simulink model "ACC_scenario_003_cs"  (Simulink 7.6)
settings: Solver=ode5  SolverMode=SingleTasking  StartTime=0.0  StopTime=inf  FixedStep=1/100  RelTol=1e-3
model workspace (MAT data in the model file):
  mbox_table : struct (value not decoded)
  model_closed = 0
  sim_status = 0
PreLoadFcn:
  | check_NUTS_defined;
  | 
  | assignin('base',['model_closed_' bdroot],false);addpath('../includes_ACC');
InitFcn:
  | PreScanExperimentInitFcn(bdroot)
StopFcn:
  | PreScanExperimentStopFcn(bdroot)
undefined symbols (not defined in the file): DiscIntNeverNeededParam, x_ini_VRML, y_ini_VRML, z_ini_VRML
libraries: PreScanSimulinkLibrary x55, simulink x26, PreScanUsefulBlocks x17, simulink_extras x15, simulink_need_slupdate x1
(part 1 of 28)
CloseFcn:
  | evalin('base',['clear model_closed_' bdroot]);
settings Model: Creator=PRESCAN  LastModifiedBy=buildbot
settings OptimizationCC: InitFltsAndDblsToZero=on
settings DebuggingCC: SaveWithDisabledLinksMsg=none  SaveWithParameterizedLinksMsg=none  SignalResolutionControl=TryResolveAllWithWarning  SolverPrmCheckMsg=none  MultiTaskDSMMsg=warning  MultiTaskCondExecSysMsg=none
settings SFSimCC: SimParseCustomCode=off
settings RTWCC: IncludeERTFirstTime=on
settings GRTTargetCC: TargetFcnLib=ansi_tfl_tmw.mat

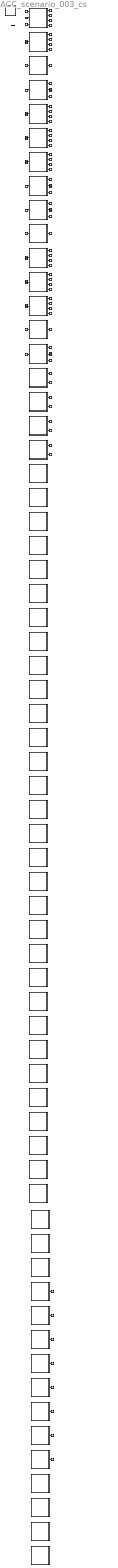
== system ACC_scenario_003_cs ==
  note: Demo Help
  note: Regenerate
  note: Record for Replay
  note: Open Useful Blocks
  note: PreScan Options
  note: Version:
  note: 7.4.0
  note: Author(s):
  note: TASS International
  note: Descriptive text:
  note: ACC test scenario
  note: ID
      |
      | 1
      | 3
      | 5
      | 383
      | 385
      | 386
      | 387
      | 388
      | 389
      | 390
      | 391
      | 392
      | 393
      | 724
      | 728
      | 380
      | 382
      | 381
      | 384
      | 403
      | 405
      | 407
      | 408
      | 409
      | 410
      | 411
      | 412
      | 413
      | 414
      | 415
      | 416
      | 417
      | 418
      | 419
      | 420
      | 421
      | 436
      | 437
      | 438
      | 439
      | 440
      | 441
      | 442
      | 443
      | 444
      | 445
      | 446
      | 447
      | 448
      | 449
      | 450
      | 451
      | 452
      | 628
      | 629
      | 612
      | 631
      | 630
      | 633
      | 632
      | 635
      | 634
      | 637
      | 636
      | 639
      | 640
      | 641
      | 642
      | 643
      | 644
      | 645
      | 716
      | 717
      | 718
      | 719
      | 592
      | 613
  note: OBU-Beacon ID
  note: TypeID
      |
      | 1
      | 1
      | 5
      | 2
      | 1
      | 1
      | 3
      | 3
      | 3
      | 7
      | 1
      | 1
      | 1
      | 3
      | 3
      | 15
      | 15
      | 15
      | 15
      | 15
      | 15
      | 15
      | 15
      | 15
      | 15
      | 15
      | 15
      | 15
      | 15
      | 15
      | 15
      | 15
      | 15
      | 15
      | 15
      | 15
      | 15
      | 15
      | 15
      | 15
      | 15
      | 15
      | 15
      | 15
      | 15
      | 15
      | 15
      | 15
      | 15
      | 15
      | 15
      | 15
      | 15
      | 15
      | 15
      | 15
      | 15
      | 15
      | 15
      | 15
      | 15
      | 15
      | 15
      | 15
      | 15
      | 15
      | 15
      | 15
      | 15
      | 15
      | 15
      | 15
      | 15
      | 15
      | 15
      | 15
      | 15
  note: TypeTag
      |
      | Car
      | Car
      | Cal element
      | Motor
      | Car
      | Car
      | Truck\Bus
      | Truck\Bus
      | Truck\Bus
      | Unknown
      | Car
      | Car
      | Car
      | Truck\Bus
      | Truck\Bus
      | Anim traffic sign
      | Anim traffic sign
      | Anim traffic sign
      | Anim traffic sign
      | Anim traffic sign
      | Anim traffic sign
      | Anim traffic sign
      | Anim traffic sign
      | Anim traffic sign
      | Anim traffic sign
      | Anim traffic sign
      | Anim traffic sign
      | Anim traffic sign
      | Anim traffic sign
      | Anim traffic sign
      | Anim traffic sign
      | Anim traffic sign
      | Anim traffic sign
      | Anim traffic sign
      | Anim traffic sign
      | Anim traffic sign
      | Anim traffic sign
      | Anim traffic sign
      | Anim traffic sign
      | Anim traffic sign
      | Anim traffic sign
      | Anim traffic sign
      | Anim traffic sign
      | Anim traffic sign
      | Anim traffic sign
      | Anim traffic sign
      | Anim traffic sign
      | Anim traffic sign
      | Anim traffic sign
      | Anim traffic sign
      | Anim traffic sign
      | Anim traffic sign
      | Anim traffic sign
      | Anim traffic sign
      | Anim traffic sign
      | Anim traffic sign
      | Anim traffic sign
      | Anim traffic sign
      | Anim traffic sign
      | Anim traffic sign
      | Anim traffic sign
      | Anim traffic sign
      | Anim traffic sign
      | Anim traffic sign
      | Anim traffic sign
      | Anim traffic sign
      | Anim traffic sign
      | Anim traffic sign
      | Anim traffic sign
      | Anim traffic sign
      | Anim traffic sign
      | Anim traffic sign
      | Anim traffic sign
      | Anim traffic sign
      | Anim traffic sign
      | Anim traffic sign
      | Anim traffic sign
  note: SD
      |
      | Y
      | Y
      | Y
      | Y
      | Y
      | Y
      | Y
      | Y
      | Y
      | Y
      | Y
      | Y
      | Y
      | Y
      | Y
      | N
      | N
      | N
      | N
      | N
      | N
      | N
      | N
      | N
      | N
      | N
      | N
      | N
      | N
      | N
      | N
      | N
      | N
      | N
      | N
      | N
      | N
      | N
      | N
      | N
      | N
      | N
      | N
      | N
      | N
      | N
      | N
      | N
      | N
      | N
      | N
      | N
      | N
      | N
      | N
      | Y
      | Y
      | Y
      | Y
      | Y
      | Y
      | Y
      | Y
      | Y
      | Y
      | N
      | N
      | N
      | N
      | N
      | N
      | N
      | N
      | N
      | N
      | Y
      | Y
  note: CD
      |
      | N
      | N
      | N
      | N
      | N
      | N
      | N
      | N
      | N
      | N
      | N
      | N
      | N
      | N
      | N
      | N
      | N
      | N
      | N
      | N
      | N
      | N
      | N
      | N
      | N
      | N
      | N
      | N
      | N
      | N
      | N
      | N
      | N
      | N
      | N
      | N
      | N
      | N
      | N
      | N
      | N
      | N
      | N
      | N
      | N
      | N
      | N
      | N
      | N
      | N
      | N
      | N
      | N
      | N
      | N
      | N
      | N
      | N
      | N
      | N
      | N
      | N
      | N
      | N
      | N
      | N
      | N
      | N
      | N
      | N
      | N
      | N
      | N
      | N
      | N
      | N
      | N
  note: Name
      |
      | Audi_A8_1
      | BMW_X5_1
      | Sphere_1
      | Honda_Pan_European_1
      | Toyota_Yaris_1
      | Toyota_Previa_1
      | Nissan_Cabstar_1
      | Actros_2541_1
      | Actros_1860_1
      | ContainerTrailer_DoubleAxle_1
      | BMW_X5_2
      | Citroen_C3_1
      | Fiat_Bravo_1
      | Actros_2541_2
      | Actros_2541_3
      | HighwayOverhang_1
      | HighwayOverhang_2
      | HighwayOverhang_3
      | HighwayOverhang_4
      | Lightpost_19
      | Lightpost_21
      | Lightpost_23
      | Lightpost_24
      | Lightpost_25
      | Lightpost_26
      | Lightpost_27
      | Lightpost_28
      | Lightpost_29
      | Lightpost_30
      | Lightpost_31
      | Lightpost_32
      | Lightpost_33
      | Lightpost_34
      | Lightpost_35
      | Lightpost_36
      | Lightpost_37
      | Lightpost_52
      | Lightpost_53
      | Lightpost_54
      | Lightpost_55
      | Lightpost_56
      | Lightpost_57
      | Lightpost_58
      | Lightpost_59
      | Lightpost_60
      | Lightpost_61
      | Lightpost_62
      | Lightpost_63
      | Lightpost_64
      | Lightpost_65
      | Lightpost_66
      | Lightpost_67
      | Lightpost_68
      | HighwayOverhang_5
      | HighwayOverhang_6
      | InformationBoard_15
      | InformationBoard_16
      | InformationBoard_17
      | InformationBoard_18
      | InformationBoard_19
      | InformationBoard_20
      | InformationBoard_21
      | InformationBoard_22
      | InformationBoard_23
      | InformationBoard_24
      | HighwayOverhang_7
      | HighwayOverhang_8
      | HighwayOverhang_9
      | HighwayOverhang_10
      | HighwayOverhang_11
      | HighwayOverhang_12
      | Lightpost_1
      | Lightpost_2
      | Lightpost_3
      | Lightpost_4
      | InformationBoard_1
      | InformationBoard_2
  note: Num ID OBU-Beacon ID Type ID Type Tag SD CD Name
  Actros_1860_1 : Subsystem  Tag=WorldObjectSubSystem  InitFcn=|
      | UniqueId = '669';
      | UniqueId = '731';
      mask Display: image(imread('Actros_1860.png'));color('white');text(0,0,' ID=389\n')
  Actros_2541_1 : Subsystem  Tag=WorldObjectSubSystem  InitFcn=UniqueId = '730';
      mask Display: image(imread('Actros_2541.png'));color('white');text(0,0,' ID=388\n')
  Actros_2541_2 : Subsystem  Tag=WorldObjectSubSystem  InitFcn=UniqueId = '988';
      mask Display: image(imread('Actros_2541.png'));color('white');text(0,0,' ID=724\n')
  Actros_2541_3 : Subsystem  Tag=WorldObjectSubSystem  InitFcn=UniqueId = '993';
      mask Display: image(imread('Actros_2541.png'));color('white');text(0,0,' ID=728\n')
  Actuators : DataStoreMemory  Tag=Actuators  DataStoreName=SimCoreActuators  InitialValue=zeros(3337,1)
      settings: DeleteFcn=PreScanBlockDeleteFcn;
  Audi_A8_1 : Subsystem  Tag=WorldObjectSubSystem  InitFcn=UniqueId = '13';
      mask Display: image(imread('Audi_A8.jpg'));color('white');text(0,0,' ID=1\n')
  BMW_X5_1 : Subsystem  Tag=WorldObjectSubSystem  InitFcn=UniqueId = '18';
      mask Display: image(imread('BMW_X5.jpg'));color('white');text(0,0,' ID=3\n')
  BMW_X5_2 : Subsystem  Tag=WorldObjectSubSystem  InitFcn=UniqueId = '740';
      mask Display: image(imread('BMW_X5.jpg'));color('white');text(0,0,' ID=391\n')
  Citroen_C3_1 : Subsystem  Tag=WorldObjectSubSystem  InitFcn=|
      | UniqueId = '642';
      | UniqueId = '741';
      mask Display: image(imread('Citroen_C3.jpg'));color('white');text(0,0,' ID=392\n')
  ContainerTrailer_DoubleAxle_1 : Subsystem  Tag=WorldObjectSubSystem  InitFcn=|
      | UniqueId = '670';
      | UniqueId = '732';
      mask Display: image(imread('ContainerTrailer_DoubleAxle.jpg'));color('white');text(0,0,' ID=390\n')
  Fiat_Bravo_1 : Subsystem  Tag=WorldObjectSubSystem  InitFcn=|
      | UniqueId = '645';
      | UniqueId = '742';
      mask Display: image(imread('Fiat_Bravo.jpg'));color('white');text(0,0,' ID=393\n')
  GetSensors : Subsystem  Tag=GetSensors  Priority=2  mask(PreScan Simulation Core Block){NrOfSensors=120, TimeStep=1/100}
      mask prompts: NrOfSensors "Number of sensor values"; TimeStep "Timestep [s]"
      mask help:
      | Please do not remove this block from the PreScan compilation sheet,
      | as it contains essential configuration parameters for a PreScan simulation.
      settings: DeleteFcn=PreScanBlockDeleteFcn;
  HighwayOverhang_1 : Subsystem  Tag=WorldObjectSubSystem  InitFcn=UniqueId = '431';
      mask Display: image(imread('HighwayOverhang.jpg'));color('white');text(0,0,' ID=380\n')
  HighwayOverhang_10 : Subsystem  Tag=WorldObjectSubSystem  InitFcn=UniqueId = '878';
      mask Display: image(imread('HighwayOverhang.jpg'));color('white');text(0,0,' ID=643\n')
  HighwayOverhang_11 : Subsystem  Tag=WorldObjectSubSystem  InitFcn=UniqueId = '891';
      mask Display: image(imread('HighwayOverhang.jpg'));color('white');text(0,0,' ID=644\n')
  HighwayOverhang_12 : Subsystem  Tag=WorldObjectSubSystem  InitFcn=UniqueId = '894';
      mask Display: image(imread('HighwayOverhang.jpg'));color('white');text(0,0,' ID=645\n')
  HighwayOverhang_2 : Subsystem  Tag=WorldObjectSubSystem  InitFcn=UniqueId = '432';
      mask Display: image(imread('HighwayOverhang.jpg'));color('white');text(0,0,' ID=382\n')
  HighwayOverhang_3 : Subsystem  Tag=WorldObjectSubSystem  InitFcn=UniqueId = '440';
      mask Display: image(imread('HighwayOverhang.jpg'));color('white');text(0,0,' ID=381\n')
  HighwayOverhang_4 : Subsystem  Tag=WorldObjectSubSystem  InitFcn=UniqueId = '441';
      mask Display: image(imread('HighwayOverhang.jpg'));color('white');text(0,0,' ID=384\n')
  HighwayOverhang_5 : Subsystem  Tag=WorldObjectSubSystem  InitFcn=UniqueId = '843';
      mask Display: image(imread('HighwayOverhang.jpg'));color('white');text(0,0,' ID=628\n')
  HighwayOverhang_6 : Subsystem  Tag=WorldObjectSubSystem  InitFcn=UniqueId = '846';
      mask Display: image(imread('HighwayOverhang.jpg'));color('white');text(0,0,' ID=629\n')
  HighwayOverhang_7 : Subsystem  Tag=WorldObjectSubSystem  InitFcn=UniqueId = '867';
      mask Display: image(imread('HighwayOverhang.jpg'));color('white');text(0,0,' ID=640\n')
  HighwayOverhang_8 : Subsystem  Tag=WorldObjectSubSystem  InitFcn=UniqueId = '870';
      mask Display: image(imread('HighwayOverhang.jpg'));color('white');text(0,0,' ID=641\n')
  HighwayOverhang_9 : Subsystem  Tag=WorldObjectSubSystem  InitFcn=UniqueId = '875';
      mask Display: image(imread('HighwayOverhang.jpg'));color('white');text(0,0,' ID=642\n')
  Honda_Pan_European_1 : Subsystem  Tag=WorldObjectSubSystem  InitFcn=|
      | UniqueId = '654';
      | UniqueId = '718';
      mask Display: image(imread('Honda_Pan_European.jpg'));color('white');text(0,0,' ID=383\n')
  Lightpost_1 : Subsystem  Tag=WorldObjectSubSystem  InitFcn=UniqueId = '976';
      mask Display: image(imread('Lightpost.jpg'));color('white');text(0,0,' ID=716\n')
  Lightpost_19 : Subsystem  Tag=WorldObjectSubSystem  InitFcn=UniqueId = '467';
      mask Display: image(imread('Lightpost.jpg'));color('white');text(0,0,' ID=403\n')
  Lightpost_2 : Subsystem  Tag=WorldObjectSubSystem  InitFcn=UniqueId = '977';
      mask Display: image(imread('Lightpost.jpg'));color('white');text(0,0,' ID=717\n')
  Lightpost_21 : Subsystem  Tag=WorldObjectSubSystem  InitFcn=UniqueId = '469';
      mask Display: image(imread('Lightpost.jpg'));color('white');text(0,0,' ID=405\n')
  Lightpost_23 : Subsystem  Tag=WorldObjectSubSystem  InitFcn=UniqueId = '471';
      mask Display: image(imread('Lightpost.jpg'));color('white');text(0,0,' ID=407\n')
  Lightpost_24 : Subsystem  Tag=WorldObjectSubSystem  InitFcn=UniqueId = '472';
      mask Display: image(imread('Lightpost.jpg'));color('white');text(0,0,' ID=408\n')
  Lightpost_25 : Subsystem  Tag=WorldObjectSubSystem  InitFcn=UniqueId = '473';
      mask Display: image(imread('Lightpost.jpg'));color('white');text(0,0,' ID=409\n')
  Lightpost_26 : Subsystem  Tag=WorldObjectSubSystem  InitFcn=UniqueId = '474';
      mask Display: image(imread('Lightpost.jpg'));color('white');text(0,0,' ID=410\n')
  Lightpost_27 : Subsystem  Tag=WorldObjectSubSystem  InitFcn=UniqueId = '475';
      mask Display: image(imread('Lightpost.jpg'));color('white');text(0,0,' ID=411\n')
  Lightpost_28 : Subsystem  Tag=WorldObjectSubSystem  InitFcn=UniqueId = '476';
      mask Display: image(imread('Lightpost.jpg'));color('white');text(0,0,' ID=412\n')
  Lightpost_29 : Subsystem  Tag=WorldObjectSubSystem  InitFcn=UniqueId = '477';
      mask Display: image(imread('Lightpost.jpg'));color('white');text(0,0,' ID=413\n')
  Lightpost_3 : Subsystem  Tag=WorldObjectSubSystem  InitFcn=UniqueId = '978';
      mask Display: image(imread('Lightpost.jpg'));color('white');text(0,0,' ID=718\n')
  Lightpost_30 : Subsystem  Tag=WorldObjectSubSystem  InitFcn=UniqueId = '478';
      mask Display: image(imread('Lightpost.jpg'));color('white');text(0,0,' ID=414\n')
  Lightpost_31 : Subsystem  Tag=WorldObjectSubSystem  InitFcn=UniqueId = '479';
      mask Display: image(imread('Lightpost.jpg'));color('white');text(0,0,' ID=415\n')
  Lightpost_32 : Subsystem  Tag=WorldObjectSubSystem  InitFcn=UniqueId = '480';
      mask Display: image(imread('Lightpost.jpg'));color('white');text(0,0,' ID=416\n')
  Lightpost_33 : Subsystem  Tag=WorldObjectSubSystem  InitFcn=UniqueId = '481';
      mask Display: image(imread('Lightpost.jpg'));color('white');text(0,0,' ID=417\n')
  Lightpost_34 : Subsystem  Tag=WorldObjectSubSystem  InitFcn=UniqueId = '482';
      mask Display: image(imread('Lightpost.jpg'));color('white');text(0,0,' ID=418\n')
  Lightpost_35 : Subsystem  Tag=WorldObjectSubSystem  InitFcn=UniqueId = '483';
      mask Display: image(imread('Lightpost.jpg'));color('white');text(0,0,' ID=419\n')
  Lightpost_36 : Subsystem  Tag=WorldObjectSubSystem  InitFcn=UniqueId = '484';
      mask Display: image(imread('Lightpost.jpg'));color('white');text(0,0,' ID=420\n')
  Lightpost_37 : Subsystem  Tag=WorldObjectSubSystem  InitFcn=UniqueId = '485';
      mask Display: image(imread('Lightpost.jpg'));color('white');text(0,0,' ID=421\n')
  Lightpost_4 : Subsystem  Tag=WorldObjectSubSystem  InitFcn=UniqueId = '979';
      mask Display: image(imread('Lightpost.jpg'));color('white');text(0,0,' ID=719\n')
  Lightpost_52 : Subsystem  Tag=WorldObjectSubSystem  InitFcn=UniqueId = '503';
      mask Display: image(imread('Lightpost.jpg'));color('white');text(0,0,' ID=436\n')
  Lightpost_53 : Subsystem  Tag=WorldObjectSubSystem  InitFcn=UniqueId = '504';
      mask Display: image(imread('Lightpost.jpg'));color('white');text(0,0,' ID=437\n')
  Lightpost_54 : Subsystem  Tag=WorldObjectSubSystem  InitFcn=UniqueId = '505';
      mask Display: image(imread('Lightpost.jpg'));color('white');text(0,0,' ID=438\n')
  Lightpost_55 : Subsystem  Tag=WorldObjectSubSystem  InitFcn=UniqueId = '506';
      mask Display: image(imread('Lightpost.jpg'));color('white');text(0,0,' ID=439\n')
  Lightpost_56 : Subsystem  Tag=WorldObjectSubSystem  InitFcn=UniqueId = '507';
      mask Display: image(imread('Lightpost.jpg'));color('white');text(0,0,' ID=440\n')
  Lightpost_57 : Subsystem  Tag=WorldObjectSubSystem  InitFcn=UniqueId = '508';
      mask Display: image(imread('Lightpost.jpg'));color('white');text(0,0,' ID=441\n')
  Lightpost_58 : Subsystem  Tag=WorldObjectSubSystem  InitFcn=UniqueId = '509';
      mask Display: image(imread('Lightpost.jpg'));color('white');text(0,0,' ID=442\n')
  Lightpost_59 : Subsystem  Tag=WorldObjectSubSystem  InitFcn=UniqueId = '510';
      mask Display: image(imread('Lightpost.jpg'));color('white');text(0,0,' ID=443\n')
  Lightpost_60 : Subsystem  Tag=WorldObjectSubSystem  InitFcn=UniqueId = '511';
      mask Display: image(imread('Lightpost.jpg'));color('white');text(0,0,' ID=444\n')
  Lightpost_61 : Subsystem  Tag=WorldObjectSubSystem  InitFcn=UniqueId = '512';
      mask Display: image(imread('Lightpost.jpg'));color('white');text(0,0,' ID=445\n')
  Lightpost_62 : Subsystem  Tag=WorldObjectSubSystem  InitFcn=UniqueId = '513';
      mask Display: image(imread('Lightpost.jpg'));color('white');text(0,0,' ID=446\n')
  Lightpost_63 : Subsystem  Tag=WorldObjectSubSystem  InitFcn=UniqueId = '514';
      mask Display: image(imread('Lightpost.jpg'));color('white');text(0,0,' ID=447\n')
  Lightpost_64 : Subsystem  Tag=WorldObjectSubSystem  InitFcn=UniqueId = '515';
      mask Display: image(imread('Lightpost.jpg'));color('white');text(0,0,' ID=448\n')
  Lightpost_65 : Subsystem  Tag=WorldObjectSubSystem  InitFcn=UniqueId = '516';
      mask Display: image(imread('Lightpost.jpg'));color('white');text(0,0,' ID=449\n')
  Lightpost_66 : Subsystem  Tag=WorldObjectSubSystem  InitFcn=UniqueId = '517';
      mask Display: image(imread('Lightpost.jpg'));color('white');text(0,0,' ID=450\n')
  Lightpost_67 : Subsystem  Tag=WorldObjectSubSystem  InitFcn=UniqueId = '518';
      mask Display: image(imread('Lightpost.jpg'));color('white');text(0,0,' ID=451\n')
  Lightpost_68 : Subsystem  Tag=WorldObjectSubSystem  InitFcn=UniqueId = '519';
      mask Display: image(imread('Lightpost.jpg'));color('white');text(0,0,' ID=452\n')
  Nissan_Cabstar_1 : Subsystem  Tag=WorldObjectSubSystem  InitFcn=|
      | UniqueId = '674';
      | UniqueId = '729';
      mask Display: image(imread('Nissan_Cabstar.jpg'));color('white');text(0,0,' ID=387\n')
  SELF_Actros_1860_1 : Subsystem  Tag=SELF  Priority=3  mask(PreScan Source Block: SELF){PortName=SELF_Actros_1860_1, PortIndex=64, PortWidth=8, TimeStep=1/100}
      mask prompts: PortName "Auto-Assigned: Port Name [-]"; PortIndex "Auto-Assigned: Port Index [-]"; PortWidth "Auto-Assigned: Port Dimensions"; TimeStep "Auto-Assigned: Upload Period [s]"
      mask help:
      | The SELF port shows (state) information of the actor itself. The outputs are position in the experiment's axis system and the GPS coordinates, and the object's absolute velocity and compass heading.
      |
      | For more information, please click Help.
      settings: DeleteFcn=PreScanBlockDeleteFcn;
      mask Help: eval('openHelp(462)')
      mask Display: image(imread('GPS.png'))
  SELF_Actros_2541_1 : Subsystem  Tag=SELF  Priority=3  mask(PreScan Source Block: SELF){PortName=SELF_Actros_2541_1, PortIndex=56, PortWidth=8, TimeStep=1/100}
      mask prompts: PortName "Auto-Assigned: Port Name [-]"; PortIndex "Auto-Assigned: Port Index [-]"; PortWidth "Auto-Assigned: Port Dimensions"; TimeStep "Auto-Assigned: Upload Period [s]"
      mask help:
      | The SELF port shows (state) information of the actor itself. The outputs are position in the experiment's axis system and the GPS coordinates, and the object's absolute velocity and compass heading.
      |
      | For more information, please click Help.
      settings: DeleteFcn=PreScanBlockDeleteFcn;
      mask Help: eval('openHelp(462)')
      mask Display: image(imread('GPS.png'))
  SELF_Actros_2541_2 : Subsystem  Tag=SELF  Priority=3  mask(PreScan Source Block: SELF){PortName=SELF_Actros_2541_2, PortIndex=104, PortWidth=8, TimeStep=1/100}
      mask prompts: PortName "Auto-Assigned: Port Name [-]"; PortIndex "Auto-Assigned: Port Index [-]"; PortWidth "Auto-Assigned: Port Dimensions"; TimeStep "Auto-Assigned: Upload Period [s]"
      mask help:
      | The SELF port shows (state) information of the actor itself. The outputs are position in the experiment's axis system and the GPS coordinates, and the object's absolute velocity and compass heading.
      |
      | For more information, please click Help.
      settings: DeleteFcn=PreScanBlockDeleteFcn;
      mask Help: eval('openHelp(462)')
      mask Display: image(imread('GPS.png'))
  SELF_Actros_2541_3 : Subsystem  Tag=SELF  Priority=3  mask(PreScan Source Block: SELF){PortName=SELF_Actros_2541_3, PortIndex=112, PortWidth=8, TimeStep=1/100}
      mask prompts: PortName "Auto-Assigned: Port Name [-]"; PortIndex "Auto-Assigned: Port Index [-]"; PortWidth "Auto-Assigned: Port Dimensions"; TimeStep "Auto-Assigned: Upload Period [s]"
      mask help:
      | The SELF port shows (state) information of the actor itself. The outputs are position in the experiment's axis system and the GPS coordinates, and the object's absolute velocity and compass heading.
      |
      | For more information, please click Help.
      settings: DeleteFcn=PreScanBlockDeleteFcn;
      mask Help: eval('openHelp(462)')
      mask Display: image(imread('GPS.png'))
  SELF_Audi_A8_1 : Subsystem  Tag=SELF  Priority=3  mask(PreScan Source Block: SELF){PortName=SELF_Audi_A8_1, PortIndex=0, PortWidth=8, TimeStep=1/100}
      mask prompts: PortName "Auto-Assigned: Port Name [-]"; PortIndex "Auto-Assigned: Port Index [-]"; PortWidth "Auto-Assigned: Port Dimensions"; TimeStep "Auto-Assigned: Upload Period [s]"
      mask help:
      | The SELF port shows (state) information of the actor itself. The outputs are position in the experiment's axis system and the GPS coordinates, and the object's absolute velocity and compass heading.
      |
      | For more information, please click Help.
      settings: DeleteFcn=PreScanBlockDeleteFcn;
      mask Help: eval('openHelp(462)')
      mask Display: image(imread('GPS.png'))
  SELF_BMW_X5_1 : Subsystem  Tag=SELF  Priority=3  mask(PreScan Source Block: SELF){PortName=SELF_BMW_X5_1, PortIndex=8, PortWidth=8, TimeStep=1/100}
      mask prompts: PortName "Auto-Assigned: Port Name [-]"; PortIndex "Auto-Assigned: Port Index [-]"; PortWidth "Auto-Assigned: Port Dimensions"; TimeStep "Auto-Assigned: Upload Period [s]"
      mask help:
      | The SELF port shows (state) information of the actor itself. The outputs are position in the experiment's axis system and the GPS coordinates, and the object's absolute velocity and compass heading.
      |
      | For more information, please click Help.
      settings: DeleteFcn=PreScanBlockDeleteFcn;
      mask Help: eval('openHelp(462)')
      mask Display: image(imread('GPS.png'))
  SELF_BMW_X5_2 : Subsystem  Tag=SELF  Priority=3  mask(PreScan Source Block: SELF){PortName=SELF_BMW_X5_2, PortIndex=80, PortWidth=8, TimeStep=1/100}
      mask prompts: PortName "Auto-Assigned: Port Name [-]"; PortIndex "Auto-Assigned: Port Index [-]"; PortWidth "Auto-Assigned: Port Dimensions"; TimeStep "Auto-Assigned: Upload Period [s]"
      mask help:
      | The SELF port shows (state) information of the actor itself. The outputs are position in the experiment's axis system and the GPS coordinates, and the object's absolute velocity and compass heading.
      |
      | For more information, please click Help.
      settings: DeleteFcn=PreScanBlockDeleteFcn;
      mask Help: eval('openHelp(462)')
      mask Display: image(imread('GPS.png'))
  SELF_Citroen_C3_1 : Subsystem  Tag=SELF  Priority=3  mask(PreScan Source Block: SELF){PortName=SELF_Citroen_C3_1, PortIndex=88, PortWidth=8, TimeStep=1/100}
      mask prompts: PortName "Auto-Assigned: Port Name [-]"; PortIndex "Auto-Assigned: Port Index [-]"; PortWidth "Auto-Assigned: Port Dimensions"; TimeStep "Auto-Assigned: Upload Period [s]"
      mask help:
      | The SELF port shows (state) information of the actor itself. The outputs are position in the experiment's axis system and the GPS coordinates, and the object's absolute velocity and compass heading.
      |
      | For more information, please click Help.
      settings: DeleteFcn=PreScanBlockDeleteFcn;
      mask Help: eval('openHelp(462)')
      mask Display: image(imread('GPS.png'))
  SELF_ContainerTrailer_DoubleAxle_1 : Subsystem  Tag=SELF  Priority=3  mask(PreScan Source Block: SELF){PortName=SELF_ContainerTrailer_DoubleAxle_1, PortIndex=72, PortWidth=8, TimeStep=1/100}
      mask prompts: PortName "Auto-Assigned: Port Name [-]"; PortIndex "Auto-Assigned: Port Index [-]"; PortWidth "Auto-Assigned: Port Dimensions"; TimeStep "Auto-Assigned: Upload Period [s]"
      mask help:
      | The SELF port shows (state) information of the actor itself. The outputs are position in the experiment's axis system and the GPS coordinates, and the object's absolute velocity and compass heading.
      |
      | For more information, please click Help.
      settings: DeleteFcn=PreScanBlockDeleteFcn;
      mask Help: eval('openHelp(462)')
      mask Display: image(imread('GPS.png'))
  SELF_Fiat_Bravo_1 : Subsystem  Tag=SELF  Priority=3  mask(PreScan Source Block: SELF){PortName=SELF_Fiat_Bravo_1, PortIndex=96, PortWidth=8, TimeStep=1/100}
      mask prompts: PortName "Auto-Assigned: Port Name [-]"; PortIndex "Auto-Assigned: Port Index [-]"; PortWidth "Auto-Assigned: Port Dimensions"; TimeStep "Auto-Assigned: Upload Period [s]"
      mask help:
      | The SELF port shows (state) information of the actor itself. The outputs are position in the experiment's axis system and the GPS coordinates, and the object's absolute velocity and compass heading.
      |
      | For more information, please click Help.
      settings: DeleteFcn=PreScanBlockDeleteFcn;
      mask Help: eval('openHelp(462)')
      mask Display: image(imread('GPS.png'))
  SELF_Honda_Pan_European_1 : Subsystem  Tag=SELF  Priority=3  mask(PreScan Source Block: SELF){PortName=SELF_Honda_Pan_European_1, PortIndex=24, PortWidth=8, TimeStep=1/100}
      mask prompts: PortName "Auto-Assigned: Port Name [-]"; PortIndex "Auto-Assigned: Port Index [-]"; PortWidth "Auto-Assigned: Port Dimensions"; TimeStep "Auto-Assigned: Upload Period [s]"
      mask help:
      | The SELF port shows (state) information of the actor itself. The outputs are position in the experiment's axis system and the GPS coordinates, and the object's absolute velocity and compass heading.
      |
      | For more information, please click Help.
      settings: DeleteFcn=PreScanBlockDeleteFcn;
      mask Help: eval('openHelp(462)')
      mask Display: image(imread('GPS.png'))
  SELF_Nissan_Cabstar_1 : Subsystem  Tag=SELF  Priority=3  mask(PreScan Source Block: SELF){PortName=SELF_Nissan_Cabstar_1, PortIndex=48, PortWidth=8, TimeStep=1/100}
      mask prompts: PortName "Auto-Assigned: Port Name [-]"; PortIndex "Auto-Assigned: Port Index [-]"; PortWidth "Auto-Assigned: Port Dimensions"; TimeStep "Auto-Assigned: Upload Period [s]"
      mask help:
      | The SELF port shows (state) information of the actor itself. The outputs are position in the experiment's axis system and the GPS coordinates, and the object's absolute velocity and compass heading.
      |
      | For more information, please click Help.
      settings: DeleteFcn=PreScanBlockDeleteFcn;
      mask Help: eval('openHelp(462)')
      mask Display: image(imread('GPS.png'))
  SELF_Sphere_1 : Subsystem  Tag=SELF  Priority=3  mask(PreScan Source Block: SELF){PortName=SELF_Sphere_1, PortIndex=16, PortWidth=8, TimeStep=1/100}
      mask prompts: PortName "Auto-Assigned: Port Name [-]"; PortIndex "Auto-Assigned: Port Index [-]"; PortWidth "Auto-Assigned: Port Dimensions"; TimeStep "Auto-Assigned: Upload Period [s]"
      mask help:
      | The SELF port shows (state) information of the actor itself. The outputs are position in the experiment's axis system and the GPS coordinates, and the object's absolute velocity and compass heading.
      |
      | For more information, please click Help.
      settings: DeleteFcn=PreScanBlockDeleteFcn;
      mask Help: eval('openHelp(462)')
      mask Display: image(imread('GPS.png'))
  SELF_Toyota_Previa_1 : Subsystem  Tag=SELF  Priority=3  mask(PreScan Source Block: SELF){PortName=SELF_Toyota_Previa_1, PortIndex=40, PortWidth=8, TimeStep=1/100}
      mask prompts: PortName "Auto-Assigned: Port Name [-]"; PortIndex "Auto-Assigned: Port Index [-]"; PortWidth "Auto-Assigned: Port Dimensions"; TimeStep "Auto-Assigned: Upload Period [s]"
      mask help:
      | The SELF port shows (state) information of the actor itself. The outputs are position in the experiment's axis system and the GPS coordinates, and the object's absolute velocity and compass heading.
      |
      | For more information, please click Help.
      settings: DeleteFcn=PreScanBlockDeleteFcn;
      mask Help: eval('openHelp(462)')
      mask Display: image(imread('GPS.png'))
  SELF_Toyota_Yaris_1 : Subsystem  Tag=SELF  Priority=3  mask(PreScan Source Block: SELF){PortName=SELF_Toyota_Yaris_1, PortIndex=32, PortWidth=8, TimeStep=1/100}
      mask prompts: PortName "Auto-Assigned: Port Name [-]"; PortIndex "Auto-Assigned: Port Index [-]"; PortWidth "Auto-Assigned: Port Dimensions"; TimeStep "Auto-Assigned: Upload Period [s]"
      mask help:
      | The SELF port shows (state) information of the actor itself. The outputs are position in the experiment's axis system and the GPS coordinates, and the object's absolute velocity and compass heading.
      |
      | For more information, please click Help.
      settings: DeleteFcn=PreScanBlockDeleteFcn;
      mask Help: eval('openHelp(462)')
      mask Display: image(imread('GPS.png'))
  STATE_Actros_1860_1 : Subsystem  Tag=State  Priority=0  mask(PreScan Sink Block: State){x0=700, y0=12, z0=0.7, Roll0=0, Pitch0=0, Yaw0=180, TimeStep=1/100}
      mask prompts: x0 "Ini x-position VEHICLE wrt GLOBAL axis system [m]"; y0 "Ini y-position VEHICLE wrt GLOBAL axis system [m]"; z0 "Ini z-position VEHICLE wrt GLOBAL axis system [m]"; Roll0 "Ini roll-angle BODY wrt VEHICLE axis system [deg]"; Pitch0 "Ini pitch-angle BODY wrt VEHICLE axis system [deg]"; Yaw0 "Ini yaw-angle VEHICLE wrt GLOBAL axis system [deg]"; TimeStep "Time Step [s]"
      mask help:
      | The Actor State contains a vector signal of size (9x1), containing the following signals:
      |
      | -  Position                  (x,y,z)                                     [m]
      | -  Velocity                  (vx,vy,vz)                               [m/s]
      | -  Angles                    (Roll, Pitch, Yaw)                   [rad]
      |
      | The Actor state is defined in the global axis system.
      | The state is superponed on the Initial position defined in the GUI.
      |
      | For more information, please click Help.
      settings: DeleteFcn=PreScanBlockDeleteFcn;
      mask Help: eval('openHelp(222)')
      mask Display: image(imread('State.png'))
  STATE_Actros_2541_1 : Subsystem  Tag=State  Priority=0  mask(PreScan Sink Block: State){x0=600, y0=12.000000000000012, z0=0.6, Roll0=0, Pitch0=0, Yaw0=180, TimeStep=1/100}
      mask prompts: x0 "Ini x-position VEHICLE wrt GLOBAL axis system [m]"; y0 "Ini y-position VEHICLE wrt GLOBAL axis system [m]"; z0 "Ini z-position VEHICLE wrt GLOBAL axis system [m]"; Roll0 "Ini roll-angle BODY wrt VEHICLE axis system [deg]"; Pitch0 "Ini pitch-angle BODY wrt VEHICLE axis system [deg]"; Yaw0 "Ini yaw-angle VEHICLE wrt GLOBAL axis system [deg]"; TimeStep "Time Step [s]"
      mask help:
      | The Actor State contains a vector signal of size (9x1), containing the following signals:
      |
      | -  Position                  (x,y,z)                                     [m]
      | -  Velocity                  (vx,vy,vz)                               [m/s]
      | -  Angles                    (Roll, Pitch, Yaw)                   [rad]
      |
      | The Actor state is defined in the global axis system.
      | The state is superponed on the Initial position defined in the GUI.
      |
      | For more information, please click Help.
      settings: DeleteFcn=PreScanBlockDeleteFcn;
      mask Help: eval('openHelp(222)')
      mask Display: image(imread('State.png'))
  STATE_Actros_2541_2 : Subsystem  Tag=State  Priority=0  mask(PreScan Sink Block: State){x0=300, y0=49, z0=0.6, Roll0=0, Pitch0=0, Yaw0=270, TimeStep=1/100}
      mask prompts: x0 "Ini x-position VEHICLE wrt GLOBAL axis system [m]"; y0 "Ini y-position VEHICLE wrt GLOBAL axis system [m]"; z0 "Ini z-position VEHICLE wrt GLOBAL axis system [m]"; Roll0 "Ini roll-angle BODY wrt VEHICLE axis system [deg]"; Pitch0 "Ini pitch-angle BODY wrt VEHICLE axis system [deg]"; Yaw0 "Ini yaw-angle VEHICLE wrt GLOBAL axis system [deg]"; TimeStep "Time Step [s]"
      mask help:
      | The Actor State contains a vector signal of size (9x1), containing the following signals:
      |
      | -  Position                  (x,y,z)                                     [m]
      | -  Velocity                  (vx,vy,vz)                               [m/s]
      | -  Angles                    (Roll, Pitch, Yaw)                   [rad]
      |
      | The Actor state is defined in the global axis system.
      | The state is superponed on the Initial position defined in the GUI.
      |
      | For more information, please click Help.
      settings: DeleteFcn=PreScanBlockDeleteFcn;
      mask Help: eval('openHelp(222)')
      mask Display: image(imread('State.png'))
  STATE_Actros_2541_3 : Subsystem  Tag=State  Priority=0  mask(PreScan Sink Block: State){x0=509.99999999999994, y0=28.750000000000036, z0=0.6, Roll0=0, Pitch0=0, Yaw0=180, TimeStep=1/100}
      mask prompts: x0 "Ini x-position VEHICLE wrt GLOBAL axis system [m]"; y0 "Ini y-position VEHICLE wrt GLOBAL axis system [m]"; z0 "Ini z-position VEHICLE wrt GLOBAL axis system [m]"; Roll0 "Ini roll-angle BODY wrt VEHICLE axis system [deg]"; Pitch0 "Ini pitch-angle BODY wrt VEHICLE axis system [deg]"; Yaw0 "Ini yaw-angle VEHICLE wrt GLOBAL axis system [deg]"; TimeStep "Time Step [s]"
      mask help:
      | The Actor State contains a vector signal of size (9x1), containing the following signals:
      |
      | -  Position                  (x,y,z)                                     [m]
      | -  Velocity                  (vx,vy,vz)                               [m/s]
      | -  Angles                    (Roll, Pitch, Yaw)                   [rad]
      |
      | The Actor state is defined in the global axis system.
      | The state is superponed on the Initial position defined in the GUI.
      |
      | For more information, please click Help.
      settings: DeleteFcn=PreScanBlockDeleteFcn;
      mask Help: eval('openHelp(222)')
      mask Display: image(imread('State.png'))
  STATE_Audi_A8_1 : Subsystem  Tag=State  Priority=0  mask(PreScan Sink Block: State){x0=-2, y0=0, z0=0.55, Roll0=0, Pitch0=0, Yaw0=0, TimeStep=1/100}
      mask prompts: x0 "Ini x-position VEHICLE wrt GLOBAL axis system [m]"; y0 "Ini y-position VEHICLE wrt GLOBAL axis system [m]"; z0 "Ini z-position VEHICLE wrt GLOBAL axis system [m]"; Roll0 "Ini roll-angle BODY wrt VEHICLE axis system [deg]"; Pitch0 "Ini pitch-angle BODY wrt VEHICLE axis system [deg]"; Yaw0 "Ini yaw-angle VEHICLE wrt GLOBAL axis system [deg]"; TimeStep "Time Step [s]"
      mask help:
      | The Actor State contains a vector signal of size (9x1), containing the following signals:
      |
      | -  Position                  (x,y,z)                                     [m]
      | -  Velocity                  (vx,vy,vz)                               [m/s]
      | -  Angles                    (Roll, Pitch, Yaw)                   [rad]
      |
      | The Actor state is defined in the global axis system.
      | The state is superponed on the Initial position defined in the GUI.
      |
      | For more information, please click Help.
      settings: DeleteFcn=PreScanBlockDeleteFcn;
      mask Help: eval('openHelp(222)')
      mask Display: image(imread('State.png'))
  STATE_BMW_X5_1 : Subsystem  Tag=State  Priority=0  mask(PreScan Sink Block: State){x0=140.86, y0=0, z0=0.69, Roll0=0, Pitch0=0, Yaw0=0, TimeStep=1/100}
      mask prompts: x0 "Ini x-position VEHICLE wrt GLOBAL axis system [m]"; y0 "Ini y-position VEHICLE wrt GLOBAL axis system [m]"; z0 "Ini z-position VEHICLE wrt GLOBAL axis system [m]"; Roll0 "Ini roll-angle BODY wrt VEHICLE axis system [deg]"; Pitch0 "Ini pitch-angle BODY wrt VEHICLE axis system [deg]"; Yaw0 "Ini yaw-angle VEHICLE wrt GLOBAL axis system [deg]"; TimeStep "Time Step [s]"
      mask help:
      | The Actor State contains a vector signal of size (9x1), containing the following signals:
      |
      | -  Position                  (x,y,z)                                     [m]
      | -  Velocity                  (vx,vy,vz)                               [m/s]
      | -  Angles                    (Roll, Pitch, Yaw)                   [rad]
      |
      | The Actor state is defined in the global axis system.
      | The state is superponed on the Initial position defined in the GUI.
      |
      | For more information, please click Help.
      settings: DeleteFcn=PreScanBlockDeleteFcn;
      mask Help: eval('openHelp(222)')
      mask Display: image(imread('State.png'))
  STATE_BMW_X5_2 : Subsystem  Tag=State  Priority=0  mask(PreScan Sink Block: State){x0=450, y0=8.50000000000003, z0=0.69, Roll0=0, Pitch0=0, Yaw0=180, TimeStep=1/100}
      mask prompts: x0 "Ini x-position VEHICLE wrt GLOBAL axis system [m]"; y0 "Ini y-position VEHICLE wrt GLOBAL axis system [m]"; z0 "Ini z-position VEHICLE wrt GLOBAL axis system [m]"; Roll0 "Ini roll-angle BODY wrt VEHICLE axis system [deg]"; Pitch0 "Ini pitch-angle BODY wrt VEHICLE axis system [deg]"; Yaw0 "Ini yaw-angle VEHICLE wrt GLOBAL axis system [deg]"; TimeStep "Time Step [s]"
      mask help:
      | The Actor State contains a vector signal of size (9x1), containing the following signals:
      |
      | -  Position                  (x,y,z)                                     [m]
      | -  Velocity                  (vx,vy,vz)                               [m/s]
      | -  Angles                    (Roll, Pitch, Yaw)                   [rad]
      |
      | The Actor state is defined in the global axis system.
      | The state is superponed on the Initial position defined in the GUI.
      |
      | For more information, please click Help.
      settings: DeleteFcn=PreScanBlockDeleteFcn;
      mask Help: eval('openHelp(222)')
      mask Display: image(imread('State.png'))
  STATE_Citroen_C3_1 : Subsystem  Tag=State  Priority=0  mask(PreScan Sink Block: State){x0=650, y0=8.5000000000000053, z0=0.58, Roll0=0, Pitch0=0, Yaw0=180, TimeStep=1/100}
      mask prompts: x0 "Ini x-position VEHICLE wrt GLOBAL axis system [m]"; y0 "Ini y-position VEHICLE wrt GLOBAL axis system [m]"; z0 "Ini z-position VEHICLE wrt GLOBAL axis system [m]"; Roll0 "Ini roll-angle BODY wrt VEHICLE axis system [deg]"; Pitch0 "Ini pitch-angle BODY wrt VEHICLE axis system [deg]"; Yaw0 "Ini yaw-angle VEHICLE wrt GLOBAL axis system [deg]"; TimeStep "Time Step [s]"
      mask help:
      | The Actor State contains a vector signal of size (9x1), containing the following signals:
      |
      | -  Position                  (x,y,z)                                     [m]
      | -  Velocity                  (vx,vy,vz)                               [m/s]
      | -  Angles                    (Roll, Pitch, Yaw)                   [rad]
      |
      | The Actor state is defined in the global axis system.
      | The state is superponed on the Initial position defined in the GUI.
      |
      | For more information, please click Help.
      settings: DeleteFcn=PreScanBlockDeleteFcn;
      mask Help: eval('openHelp(222)')
      mask Display: image(imread('State.png'))
  STATE_ContainerTrailer_DoubleAxle_1 : Subsystem  Tag=State  Priority=0  mask(PreScan Sink Block: State){x0=702.2852, y0=12, z0=1.1041, Roll0=0, Pitch0=0, Yaw0=180, TimeStep=1/100}
      mask prompts: x0 "Ini x-position VEHICLE wrt GLOBAL axis system [m]"; y0 "Ini y-position VEHICLE wrt GLOBAL axis system [m]"; z0 "Ini z-position VEHICLE wrt GLOBAL axis system [m]"; Roll0 "Ini roll-angle BODY wrt VEHICLE axis system [deg]"; Pitch0 "Ini pitch-angle BODY wrt VEHICLE axis system [deg]"; Yaw0 "Ini yaw-angle VEHICLE wrt GLOBAL axis system [deg]"; TimeStep "Time Step [s]"
      mask help:
      | The Actor State contains a vector signal of size (9x1), containing the following signals:
      |
      | -  Position                  (x,y,z)                                     [m]
      | -  Velocity                  (vx,vy,vz)                               [m/s]
      | -  Angles                    (Roll, Pitch, Yaw)                   [rad]
      |
      | The Actor state is defined in the global axis system.
      | The state is superponed on the Initial position defined in the GUI.
      |
      | For more information, please click Help.
      settings: DeleteFcn=PreScanBlockDeleteFcn;
      mask Help: eval('openHelp(222)')
      mask Display: image(imread('State.png'))
  STATE_Fiat_Bravo_1 : Subsystem  Tag=State  Priority=0  mask(PreScan Sink Block: State){x0=550, y0=8.5000000000000178, z0=0.58, Roll0=0, Pitch0=0, Yaw0=180, TimeStep=1/100}
      mask prompts: x0 "Ini x-position VEHICLE wrt GLOBAL axis system [m]"; y0 "Ini y-position VEHICLE wrt GLOBAL axis system [m]"; z0 "Ini z-position VEHICLE wrt GLOBAL axis system [m]"; Roll0 "Ini roll-angle BODY wrt VEHICLE axis system [deg]"; Pitch0 "Ini pitch-angle BODY wrt VEHICLE axis system [deg]"; Yaw0 "Ini yaw-angle VEHICLE wrt GLOBAL axis system [deg]"; TimeStep "Time Step [s]"
      mask help:
      | The Actor State contains a vector signal of size (9x1), containing the following signals:
      |
      | -  Position                  (x,y,z)                                     [m]
      | -  Velocity                  (vx,vy,vz)                               [m/s]
      | -  Angles                    (Roll, Pitch, Yaw)                   [rad]
      |
      | The Actor state is defined in the global axis system.
      | The state is superponed on the Initial position defined in the GUI.
      |
      | For more information, please click Help.